simulink model "ofdm_txrx_supermimo_coded"  (Simulink 7.7)
settings: Solver=VariableStepDiscrete  SolverMode=SingleTasking  StartTime=0.0  StopTime=2*simOnly_simLength  RelTol=1e-3
PostLoadFcn:
  | ofdm_rx_supermimo_init
InitFcn:
  | ofdm_rx_supermimo_init
StartFcn:
  | ofdm_rx_supermimo_init
values from neighbouring files (symbols this model uses):
  ActionID_AFTransmit = 31    [ofdm_tx_supermimo_init.m]
  ActionID_Disabled = 0    [ofdm_tx_supermimo_init.m]
  ActionID_SetFlagA = 62    [ofdm_tx_supermimo_init.m]
  ActionID_SetFlagB = 61    [ofdm_tx_supermimo_init.m]
  BER_RAM_init_size = 2048    [ofdm_tx_supermimo_init.m]
  channel_h = [1 0; 1 0] * 0.3    [ofdm_tx_supermimo_init.m]
  CPLength = 16    [ofdm_tx_supermimo_init.m]
  longCorr_coef_i = [-4*real(LTS) 0]    [ofdm_rx_supermimo_init.m]
  longCorr_coef_q = [4*imag(LTS) 0]    [ofdm_rx_supermimo_init.m]
  max_CP_length = 16    [ofdm_tx_supermimo_init.m]
  max_num_baseRateSymbols = 31    [ofdm_tx_supermimo_init.m]
  max_num_subcarriers = 64    [ofdm_tx_supermimo_init.m]
  max_num_trainingSymbols = 15    [ofdm_tx_supermimo_init.m]
  max_numBytes = 16384    [ofdm_tx_supermimo_init.m]
  max_OFDM_symbols = 1023    [ofdm_tx_supermimo_init.m]
  modConstellation_qam16 = .75*[1 3 -1 -3]./3    [ofdm_tx_supermimo_init.m]
  modConstellation_qam64 = 0.875*[3 1 5 7 -3 -1 -5 -7]./7    [ofdm_tx_supermimo_init.m]
  modConstellation_qpsk = [1 -1]./sqrt(2)    [ofdm_tx_supermimo_init.m]
  numBaseRateSymbols = numBaseRateSymbols *2    [ofdm_tx_supermimo_init.m]
  numSubcarriers = 64    [ofdm_tx_supermimo_init.m]
  numTrainingSymbols = 2    [ofdm_tx_supermimo_init.m]
  pktTiming_count_payloadStart = pktTiming_count_lsStart + pktTiming_ls_num*pktTiming_ls_length    [ofdm_rx_supermimo_init.m]
  preamble = preamble_scale*[shortsyms_10 longsyms_2]    [ofdm_tx_supermimo_init.m]
  preamble_ROM = preamble_I_ROM + preamble_Q_ROM*2^16    [ofdm_tx_supermimo_init.m]
  RAM_init_size = max_numBytes    (= 16384)    [ofdm_tx_supermimo_init.m]
  RxDataScrambling_Seq = [40 198 78 63 82 173 102 245 48 111 172 115 147 230 216 93 72 65 62 2 205 242 122 90 128 83 105 97 73 10 5 252]    [ofdm_tx_supermimo_init.m]
  shortSymbol_time = shortSymbol_time(1:16).'    [ofdm_tx_supermimo_init.m]
  simOnly_numSamples = length(preamble)+( (numSubcarriers+CPLength)*(numTrainingSymbols + numBaseRateSymbols + numFullRateSymbols/2) )    [ofdm_tx_supermimo_init.m]
  train = [train train]    [ofdm_tx_supermimo_init.m]
  TxDataScrambling_Seq = [40 198 78 63 82 173 102 245 48 111 172 115 147 230 216 93 72 65 62 2 205 242 122 90 128 83 105 97 73 10 5 252]    [ofdm_tx_supermimo_init.m]
undefined symbols (not defined in the file or in its folder): AntA, AntB, BaseRate, Down, gcbh, numBuffs, numBytes, Up, xlBlockLoadCallback, xlGetNormalizedPeriod, xlipgetpartsetting
libraries: xbsIndex_r4 x5430
(part 1 of 49)
settings Model: Creator=murphpo  LastModifiedBy=murphpo
settings DataIOCC: LimitDataPoints=off  SaveOutput=off  SignalLogging=off  SaveTime=off
settings OptimizationCC: InlineParams=on  InitFltsAndDblsToZero=on
settings DebuggingCC: SaveWithParameterizedLinksMsg=none  StrictBusMsg=Warning

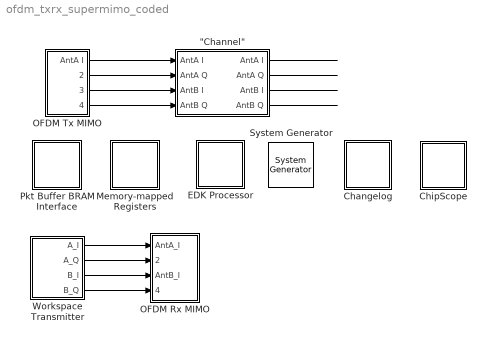
== system ofdm_txrx_supermimo_coded ==
  " System Generator" : xbsIndex_r4/ System Generator  Tag=genX  SystemSampleTime=-1  xilinxfamily=virtex6  part=xc6vlx130t  speed=-1  package=ff1156  synthesis_tool=XST  clock_wrapper=Clock Enables  directory=./OFDM_txrx_SG13_V6_v04_12bitIO  proj_type=Project Navigator  Synth_file=XST Defaults  Impl_file=ISE Defaults  testbench=off  simulink_period=1  sysclk_period=10  dcm_input_clock_period=100  incr_netlist=off  trim_vbits=Everywhere in SubSystem  dbl_ovrd=According to Block Masks  core_generation=According to Block Masks  run_coregen=off  deprecated_control=off  eval_field=0  block_type=sysgen
      settings: block_version=10.1.3
  "\"Channel\"" : Subsystem  mask{h=channel_h %[1 0; 0 0] * 0.65 % [1 0; 0 0] * 0.5, freqOffsetA=7.5e-4, freqOffsetB=0, awgnSNR=40, phNoise=-5000}
      mask prompts: h "2x2 Channel Matrix"; freqOffsetA "Freq Offset A (phase inc [0,1])"; freqOffsetB "Freq Offset B (phase inc [0,1])"; awgnSNR "SNR (dB)"; phNoise "Phase Noise (dBc @ 100Hz)"
      mask help: This block implements a simple static 2x2 channel. The one parameter sets the 2x2 channel matrix. h=[1 0;0 1] would be perfectly orthogonal channels. The norm of the matrix should be 1 to avoid overflow.
  Changelog : Subsystem
  ChipScope : Subsystem
  EDK Processor : Subsystem  LoadFcn=xlBlockLoadCallback(gcbh);  mask(Xilinx EDK Processor Block){mode=EDK pcore generation, xmp=, MemVisToProc=<qt bgcolor="#FFFFFF"><div><img src="C:/Xilinx/13.4/ISE_DS/ISE/sysgen/data/images/registerplus.gif"> &lt;&lt;FEC_Config&gt;&gt;<br></div><div><img src="C:/Xilinx/13.4/ISE_DS/ISE/sysgen/data/images/registerplus.gif"> &lt;&lt;Tx_OFDM_SymCounts&gt;&gt;<br></div><div><img src="C:/Xilinx/13.4/ISE_DS/ISE/sysgen/data/images/registerplus.gif"> &lt;&lt;Rx_OFDM_SymbolCounts&gt;&gt;<br></div><div><img src="C:/Xilinx/13.4/ISE_DS/ISE/sysgen/data/images/registerplus.gif"> &lt;&lt;TxRx_Pilots_Values&gt;&gt;<br></div><div><img src="C:/Xilinx/13.4/ISE_DS/ISE/sysgen/data/images/registerplus.gif"> &lt;&lt;TxRx_Pilots_Index&gt;&gt;<br></div><div><img src="C:/Xilinx/13.4/ISE_DS/ISE/sysgen/data/images/registerplus.gif"> &lt;&lt;TxRx_Interrupt_PktBuf_Ctrl&gt;&gt;<br></div><div><img src="C:/Xilinx/13.4/ISE_DS/ISE/sysgen/data/images/registerplus.gif"> &lt;&lt;TxRx_FFT_Scaling&gt;&gt;<br></div><div><img src="C:/Xilinx/13.4/ISE_DS/ISE/sysgen/data/images/registerplus.gif"> &lt;&lt;Tx_Start_Reset_Control&gt;&gt;<br></div><div><img src="C:/Xilinx/13.4/ISE_DS/ISE/sysgen/data/images/registerplus.gif"> &lt;&lt;Tx_ControlBits&gt;&gt;<br></div><div><img src="C:/Xilinx/13.4/ISE_DS/ISE/sysgen/data/images/registerplus.gif"> &lt;&lt;Tx_Delays&gt;&gt;<br></div><div><img src="C:/Xilinx/13.4/ISE_DS/ISE/sysgen/data/images/registerplus.gif"> &lt;&lt;Tx_Scaling&gt;&gt;<br></div><div><img src="C:/Xilinx/13.4/ISE_DS/ISE/sysgen/data/images/registerplus.gif"> &lt;&lt;pktDet_thresholds&gt;&gt;<br></div><div><img src="C:/Xilinx/13.4/ISE_DS/ISE/sysgen/data/images/registerplus.gif"> &lt;&lt;pktDet_durations&gt;&gt;<br></div><div><img src="C:/Xilinx/13.4/ISE_DS/ISE/sysgen/data/images/registerplus.gif"> &lt;&lt;pktDet_autoCorrParams&gt;&gt;<br></div><div><img src="C:/Xilinx/13.4/ISE_DS/ISE/sysgen/data/images/registerplus.gif"> &lt;&lt;pktDet_controlBits&gt;&gt;<br></div><div><img src="C:/Xilinx/13.4/ISE_DS/ISE/sysgen/data/images/registerplus.gif"> &lt;&lt;Rx_AF_TxScaling&gt;&gt;<br></div><div><img src="C:/Xilinx/13.4/ISE_DS/ISE/sysgen/data/images/registerplus.gif"> &lt;&lt;Rx_AF_Blanking&gt;&gt;<br></div><div><img src="C:/Xilinx/13.4/ISE_DS/ISE/sysgen/data/images/registerplus.gif"> &lt;&lt;Rx_PilotCalcParams&gt;&gt;<br></div><div><img src="C:/Xilinx/13.4/ISE_DS/ISE/sysgen/data/images/registerminus.gif"> &lt;&lt;Rx_Constellation_Scaling&gt;&gt;<br> <img src="C:/Xilinx/13.4/ISE_DS/ISE/sysgen/data/images/parameters.gif"> Type : Fix_32_0<br> <img src="C:/Xilinx/13.4/ISE_DS/ISE/sysgen/data/images/parameters.gif"> Addr : 0x80008048<br></div><div><img src="C:/Xilinx/13.4/ISE_DS/ISE/sysgen/data/images/registerplus.gif"> &lt;&lt;Rx_PktDet_Delay&gt;&gt;<br></div><div><img src="C:/Xilinx/13.4/ISE_DS/ISE/sysgen/data/images/registerplus.gif"> &lt;&lt;Rx_ChanEst_MinMag&gt;&gt;<br></div><div><img src="C:/Xilinx/13.4/ISE_DS/ISE/sysgen/data/images/registerplus.gif"> &lt;&lt;Rx_pktByteNums&gt;&gt;<br></div><div><img src="C:/Xilinx/13.4/ISE_DS/ISE/sysgen/data/images/registerplus.gif"> &lt;&lt;Rx_FixedPktLen&gt;&gt;<br></div><div><img src="C:/Xilinx/13.4/ISE_DS/ISE/sysgen/data/images/registerplus.gif"> &lt;&lt;Rx_PktDet_LongCorr_Thresholds&gt;&gt;<br></div><div><img src="C:/Xilinx/13.4/ISE_DS/ISE/sysgen/data/images/registerplus.gif"> &lt;&lt;Rx_PktDet_LongCorr_Params&gt;&gt;<br></div><div><img src="C:/Xilinx/13.4/ISE_DS/ISE/sysgen/data/images/registerplus.gif"> &lt;&lt;Rx_coarseCFO_correction&gt;&gt;<br></div><div><img src="C:/Xilinx/13.4/ISE_DS/ISE/sysgen/data/images/registerplus.gif"> &lt;&lt;Rx_PreCFO_PilotCalcCorrection&gt;&gt;<br></div><div><img src="C:/Xilinx/13.4/ISE_DS/ISE/sysgen/data/images/registerplus.gif"> &lt;&lt;Rx_PreCFO_Options&gt;&gt;<br></div><div><img src="C:/Xilinx/13.4/ISE_DS/ISE/sysgen/data/images/registerplus.gif"> &lt;&lt;Rx_ControlBits&gt;&gt;<br></div><div><img src="C:/Xilinx/13.4/ISE_DS/ISE/sysgen/data/images/registerplus.gif"> &lt;&lt;TxRx_AutoReply_Action3&gt;&gt;<br></div><div><img src="C:/Xilinx/13.4/ISE_DS/ISE/sysgen/data/images/registerplus.gif"> &lt;&lt;TxRx_AutoReply_Action2&gt;&gt;<br></div><div><img src="C:/Xilinx/13.4/ISE_DS/ISE/sysgen/data/images/registerplus.gif"> &lt;&lt;TxRx_AutoReply_Action1&gt;&gt;<br></div><div><img src="C:/Xilinx/13.4/ISE_DS/ISE/sysgen/data/images/registerplus.gif"> &lt;&lt;TxRx_AutoReply_Action0&gt;&gt;<br></div><div><img src="C:/Xilinx/13.4/ISE_DS/ISE/sysgen/data/images/registerplus.gif"> &lt;&lt;TxRx_AutoReply_Match5&gt;&gt;<br></div><div><img src="C:/Xilinx/13.4/ISE_DS/ISE/sysgen/data/images/registerplus.gif"> &lt;&lt;TxRx_AutoReply_Match4&gt;&gt;<br></div><div><img src="C:/Xilinx/13.4/ISE_DS/ISE/sysgen/data/images/registerplus.gif"> &lt;&lt;TxRx_AutoReply_Match3&gt;&gt;<br></div><div><img src="C:/Xilinx/13.4/ISE_DS/ISE/sysgen/data/images/registerplus.gif"> &lt;&lt;TxRx_AutoReply_Match2&gt;&gt;<br></div><div><img src="C:/Xilinx/13.4/ISE_DS/ISE/sysgen/data/images/registerplus.gif"> &lt;&lt;TxRx_AutoReply_Action5&gt;&gt;<br></div><div><img src="C:/Xilinx/13.4/ISE_DS/ISE/sysgen/data/images/registerplus.gif"> &lt;&lt;TxRx_AutoReply_Action4&gt;&gt;<br></div><div><img src="C:/Xilinx/13.4/ISE_DS/ISE/sysgen/data/images/registerplus.gif"> &lt;&lt;TxRx_AutoReply_Match1&gt;&gt;<br></div><div><img src="C:/Xilinx/13.4/ISE_DS/ISE/sysgen/data/images/registerplus.gif"> &lt;&lt;TxRx_AutoReply_Match0&gt;&gt;<br></div><div><img src="C:/Xilinx/13.4/ISE_DS/ISE/sysgen/data/images/registerplus.gif"> &lt;&lt;midPacketRSSI&gt;&gt;<br></div><div><img src="C:/Xilinx/13.4/ISE_DS/ISE/sysgen/data/images/registerplus.gif"> &lt;&lt;Tx_PktRunning&gt;&gt;<br></div><div><img src="C:/Xilinx/13.4/ISE_DS/ISE/sysgen/data/images/registerplus.gif"> &lt;&lt;Rx_pktDetEventCount&gt;&gt;<br></div><div><img src="C:/Xilinx/13.4/ISE_DS/ISE/sysgen/data/images/registerplus.gif"> &lt;&lt;Rx_coarseCFOest&gt;&gt;<br></div><div><img src="C:/Xilinx/13.4/ISE_DS/ISE/sysgen/data/images/registerplus.gif"> &lt;&lt;Rx_pilotCFOest&gt;&gt;<br></div><div><img src="C:/Xilinx/13.4/ISE_DS/ISE/sysgen/data/images/registerplus.gif"> &lt;&lt;Rx_pktDone_interruptStatus&gt;&gt;<br></div><div><img src="C:/Xilinx/13.4/ISE_DS/ISE/sysgen/data/images/registerplus.gif"> &lt;&lt;Rx_BER_TotalBits&gt;&gt;<br></div><div><img src="C:/Xilinx/13.4/ISE_DS/ISE/sysgen/data/images/registerplus.gif"> &lt;&lt;Rx_BER_Errors&gt;&gt;<br></div><div><img src="C:/Xilinx/13.4/ISE_DS/ISE/sysgen/data/images/registerplus.gif"> &lt;&lt;Rx_Gains&gt;&gt;<br></div><div><img src="C:/Xilinx/13.4/ISE_DS/ISE/sysgen/data/images/registerplus.gif"> &lt;&lt;pktDet_status&gt;&gt;<br></div><div><img src="C:/Xilinx/13.4/ISE_DS/ISE/sysgen/data/images/memoryplus.gif"> &lt;&lt;TxModulation&gt;&gt;<br></div><div><img src="C:/Xilinx/13.4/ISE_DS/ISE/sysgen/data/images/memoryplus.gif"> &lt;&lt;TxHeaderTranslate&gt;&gt;<br></div><div><img src="C:/Xilinx/13.4/ISE_DS/ISE/sysgen/data/images/memoryplus.gif"> &lt;&lt;ChannelEstimates&gt;&gt;<br></div><div><img src="C:/Xilinx/13.4/ISE_DS/ISE/sysgen/data/images/memoryplus.gif"> &lt;&lt;RxModulation&gt;&gt;<br></div><div><img src="C:/Xilinx/13.4/ISE_DS/ISE/sysgen/data/images/memoryplus.gif"> &lt;&lt;EVM_perSym&gt;&gt;<br></div><div><img src="C:/Xilinx/13.4/ISE_DS/ISE/sysgen/data/images/memoryplus.gif"> &lt;&lt;EVM_perSC&gt;&gt;<br></div><div><img src="C:/Xilinx/13.4/ISE_DS/ISE/sysgen/data/images/memoryplus.gif"> &lt;&lt;PktBufFreqOffsets&gt;&gt;<br></div></qt>, AvailableMemories=<empty>, portInterfaceTable={'exposed'=>[],'portdir'=>[],'portname'=>[],'shortname'=>[]}, bus_type_sgadvanced=, bus_type=PLB, baseaddr=0x80000000, baseaddr_lock_sgadvanced=, baseaddr_lock=off, dual_clock_sgadvanced=, dual_clock=on, reg_readback_sgadvanced=, reg_readback=on, ucf_file=, inheritDeviceType_sgadvanced=, inheritDeviceType=off, elf_file=, codebug_sgadvanced=, codebug=on, clock_name=plb, internalPortList={}, resetPolarity=0, memxtable=|, procinfo={}, memmapdirty=off, blockname=, xpsintstyle=default, has_advanced_control=0, sggui_pos=-1,-1,-1,-1, block_type=edkprocessor, block_version=2.7, sg_icon_stat=44,44,-1,-1,white,blue,0,07734,right,,[ ],[ ], sg_mask_display=|, sg_list_contents={'table'=>{'AvailableMemories'=>'popup(<empty>)'}}, sg_blockgui_xml=}
      mask memxtable=|
      | {'mladdr'=>[0.00000000000000000,1.00000000000000000,2.00000000000000000,3.00000000000000000,4.00000000000000000,5.00000000000000000,6.00000000000000000,7.00000000000000000,8.00000000000000000,9.00000000000000000,10.00000000000000000,11.00000000000000000,12.00000000000000000,13.00000000000000000,14.00000000000000000,15.00000000000000000,16.00000000000000000,17.00000000000000000,18.00000000000000000,19.00000000000000000,20.00000000000000000,21.00000000000000000,22.00000000000000000,23.00000000000000000,24.00000000000000000,25.00000000000000000,26.00000000000000000,27.00000000000000000,28.00000000000000000,29.00000000000000000,30.00000000000000000,31.00000000000000000,32.00000000000000000,33.00000000000000000,34.00000000000000000,35.00000000000000000,36.00000000000000000,37.00000000000000000,38.00000000000000000,39.00000000000000000,40.00000000000000000,0.00000000000000000,1.00000000000000000,2.00000000000000000,3.00000000000000000,4.00000000000000000,5.00000000000000000,6.00000000000000000,7.00000000000000000,8.00000000000000000,9.00000000000000000,-1.00000000000000000,-1.00000000000000000,-1.00000000000000000,-1.00000000000000000,-1.00000000000000000,-1.00000000000000000,-1.00000000000000000],'mlist'=>['ofdm_txrx_supermimo_coded/Memory-mapped
      | Registers/TxRx Registers/From Register8','ofdm_txrx_supermimo_coded/Memory-mapped
      | Registers/TxRx Registers/From Register7','ofdm_txrx_supermimo_coded/Memory-mapped
      | Registers/TxRx Registers/From Register6','ofdm_txrx_supermimo_coded/Memory-mapped
      | Registers/TxRx Registers/From Register5','ofdm_txrx_supermimo_coded/Memory-mapped
      | Registers/TxRx Registers/From Register4','ofdm_txrx_supermimo_coded/Memory-mapped
      | Registers/TxRx Registers/From Register3','ofdm_txrx_supermimo_coded/Memory-mapped
      | Registers/TxRx Registers/From Register2','ofdm_txrx_supermimo_coded/Memory-mapped
      | Registers/Tx Registers/From Register3','ofdm_txrx_supermimo_coded/Memory-mapped
      | Registers/Tx Registers/From Register2','ofdm_txrx_supermimo_coded/Memory-mapped
      | Registers/Tx Registers/From Register10','ofdm_txrx_supermimo_coded/Memory-mapped
      | Registers/Tx Registers/From Register1','ofdm_txrx_supermimo_coded/Memory-mapped
      | Registers/Rx Registers/Pkt Detector Registers/From Register4','ofdm_txrx_supermimo_coded/Memory-mapped
      | Registers/Rx Registers/Pkt Detector Registers/From Register3','ofdm_txrx_supermimo_coded/Memory-mapped
      | Registers/Rx Registers/Pkt Detector Registers/From Register2','ofdm_txrx_supermimo_coded/Memory-mapped
      | Registers/Rx Registers/Pkt Detector Registers/From Register1','ofdm_txrx_supermimo_coded/Memory-mapped
      | Registers/Rx Registers/From Register8','ofdm_txrx_supermimo_coded/Memory-mapped
      | Registers/Rx Registers/From Register7','ofdm_txrx_supermimo_coded/Memory-mapped
      | Registers/Rx Registers/From Register6','ofdm_txrx_supermimo_coded/Memory-mapped
      | Registers/Rx Registers/From Register5','ofdm_txrx_supermimo_coded/Memory-mapped
      | Registers/Rx Registers/From Register4','ofdm_txrx_supermimo_coded/Memory-mapped
      | Registers/Rx Registers/From Register3','ofdm_txrx_supermimo_coded/Memory-mapped
      | Registers/Rx Registers/From Register2','ofdm_txrx_supermimo_coded/Memory-mapped
      | Registers/Rx Registers/From Register16','ofdm_txrx_supermimo_coded/Memory-mapped
      | Registers/Rx Registers/From Register14','ofdm_txrx_supermimo_coded/Memory-mapped
      | Registers/Rx Registers/From Register13','ofdm_txrx_supermimo_coded/Memory-mapped
      | Registers/Rx Registers/From Register12','ofdm_txrx_supermimo_coded/Memory-mapped
      | Registers/Rx Registers/From Register11','ofdm_txrx_supermimo_coded/Memory-mapped
      | Registers/Rx Registers/From Register10','ofdm_txrx_supermimo_coded/Memory-mapped
      | Registers/Rx Registers/From Register','ofdm_txrx_supermimo_coded/Memory-mapped
      | Registers/AutoReply Registers/From Register9','ofdm_txrx_supermimo_coded/Memory-mapped
      | Registers/AutoReply Registers/From Register8','ofdm_txrx_supermimo_coded/Memory-mapped
      | Registers/AutoReply Registers/From Register7','ofdm_txrx_supermimo_coded/Memory-mapped
      | Registers/AutoReply Registers/From Register6','ofdm_txrx_supermimo_coded/Memory-mapped
      | Registers/AutoReply Registers/From Register5','ofdm_txrx_supermimo_coded/Memory-mapped
      | Registers/AutoReply Registers/From Register4','ofdm_txrx_supermimo_coded/Memory-mapped
      | Registers/AutoReply Registers/From Register3','ofdm_txrx_supermimo_coded/Memory-mapped
      | Registers/AutoReply Registers/From Register2','ofdm_txrx_supermimo_coded/Memory-mapped
      | Registers/AutoReply Registers/From Register11','ofdm_txrx_supermimo_coded/Memory-mapped
      | Registers/AutoReply Registers/From Register10','ofdm_txrx_supermimo_coded/Memory-mapped
      | Registers/AutoReply Registers/From Register1','ofdm_txrx_supermimo_coded/Memory-mapped
      | Registers/AutoReply Registers/From Register','ofdm_txrx_supermimo_coded/OFDM Rx MIMO/Packet_Detection/RSSI-based PktDet/Mid-Packet
      | RSSI Register/To Register2','ofdm_txrx_supermimo_coded/Memory-mapped
      | Registers/Tx Registers/To Register7','ofdm_txrx_supermimo_coded/Memory-mapped
      | Registers/Rx Registers/To Register6','ofdm_txrx_supermimo_coded/Memory-mapped
      | Registers/Rx Registers/To Register5','ofdm_txrx_supermimo_coded/Memory-mapped
      | Registers/Rx Registers/To Register4','ofdm_txrx_supermimo_coded/Memory-mapped
      | Registers/Rx Registers/To Register3','ofdm_txrx_supermimo_coded/Memory-mapped
      | Registers/Rx Registers/To Register2','ofdm_txrx_supermimo_coded/Memory-mapped
      | Registers/Rx Registers/To Register1','ofdm_txrx_supermimo_coded/Memory-mapped
      | Registers/Rx Registers/To Register','ofdm_txrx_supermimo_coded/Memory-mapped
      | Registers/Rx Registers/Pkt Detector Registers/To Register','ofdm_txrx_supermimo_coded/OFDM Tx MIMO/Training_Data/FlexibleMod/control/Tx Modulation/Shared Memory for
      | Modulation Masks/Shared Memory','ofdm_txrx_supermimo_coded/OFDM Tx MIMO/Training_Data/FlexibleMod/PktBuffer_CRC1/Packet Buffer/AutoTx
      | Header Translation/Shared Memory','ofdm_txrx_supermimo_coded/OFDM Rx MIMO/FFT & Chan Est/Channel Estimation
      | & Pilot Processing/Chan Est Buffer
      | & Mag Calc/Shared Memory','ofdm_txrx_supermimo_coded/OFDM Rx MIMO/Equalizer & Packetizer/Packet_Constructor/Modulation RAM/Modulation Masks/Shared Memory','ofdm_txrx_supermimo_coded/OFDM Rx MIMO/Equalizer & Packetizer/Packet_Constructor/EVM Calc/EVM per Symbol/Shared Memory','ofdm_txrx_supermimo_coded/OFDM Rx MIMO/Equalizer & Packetizer/Packet_Constructor/EVM Calc/EVM per Subcarrier/Shared Memory','ofdm_txrx_supermimo_coded/OFDM Rx MIMO/Coarse Freq Correction/PreSpin CFO Sel/Shared Memory'],'mlname'=>['\\'FEC_Config\\'','\\'Tx_OFDM_SymCounts\\'','\\'Rx_OFDM_SymbolCounts\\'','\\'TxRx_Pilots_Values\\'','\\'TxRx_Pilots_Index\\'','\\'TxRx_Interrupt_PktBuf_Ctrl\\'','\\'TxRx_FFT_Scaling\\'','\\'Tx_Start_Reset_Control\\'','\\'Tx_ControlBits\\'','\\'Tx_Delays\\'','\\'Tx_Scaling\\'','\\'pktDet_thresholds\\'','\\'pktDet_durations\\'','\\'pktDet_autoCorrParams\\'','\\'pktDet_controlBits\\'','\\'Rx_AF_TxScaling\\'','\\'Rx_AF_Blanking\\'','\\'Rx_PilotCalcParams\\'','\\'Rx_Constellation_Scaling\\'','\\'Rx_PktDet_Delay\\'','\\'Rx_ChanEst_MinMag\\'','\\'Rx_pktByteNums\\'','\\'Rx_FixedPktLen\\'','\\'Rx_PktDet_LongCorr_Thresholds\\'','\\'Rx_PktDet_LongCorr_Params\\'','\\'Rx_coarseCFO_correction\\'','\\'Rx_PreCFO_PilotCalcCorrection\\'','\\'Rx_PreCFO_Options\\'','\\'Rx_ControlBits\\'','\\'TxRx_AutoReply_Action3\\'','\\'TxRx_AutoReply_Action2\\'','\\'TxRx_AutoReply_Action1\\'','\\'TxRx_AutoReply_Action0\\'','\\'TxRx_AutoReply_Match5\\'','\\'TxRx_AutoReply_Match4\\'','\\'TxRx_AutoReply_Match3\\'','\\'TxRx_AutoReply_Match2\\'','\\'TxRx_AutoReply_Action5\\'','\\'TxRx_AutoReply_Action4\\'','\\'TxRx_AutoReply_Match1\\'','\\'TxRx_AutoReply_Match0\\'','\\'midPacketRSSI\\'','\\'Tx_PktRunning\\'','\\'Rx_pktDetEventCount\\'','\\'Rx_coarseCFOest\\'','\\'Rx_pilotCFOest\\'','\\'Rx_pktDone_interruptStatus\\'','\\'Rx_BER_TotalBits\\'','\\'Rx_BER_Errors\\'','\\'Rx_Gains\\'','\\'pktDet_status\\'','\\'TxModulation\\'','\\'TxHeaderTranslate\\'','\\'ChannelEstimates\\'','\\'RxModulation\\'','\\'EVM_perSym\\'','\\'EVM_perSC\\'','\\'PktBufFreqOffsets\\''],'mlstate'=>[0.00000000000000000,0.00000000000000000,0.00000000000000000,0.00000000000000000,0.00000000000000000,0.00000000000000000,0.00000000000000000,0.00000000000000000,0.00000000000000000,0.00000000000000000,0.00000000000000000,0.00000000000000000,0.00000000000000000,0.00000000000000000,0.00000000000000000,0.00000000000000000,0.00000000000000000,0.00000000000000000,1.00000000000000000,0.00000000000000000,0.00000000000000000,0.00000000000000000,0.00000000000000000,0.00000000000000000,0.00000000000000000,0.00000000000000000,0.00000000000000000,0.00000000000000000,0.00000000000000000,0.00000000000000000,0.00000000000000000,0.00000000000000000,0.00000000000000000,0.00000000000000000,0.00000000000000000,0.00000000000000000,0.00000000000000000,0.00000000000000000,0.00000000000000000,0.00000000000000000,0.00000000000000000,0.00000000000000000,0.00000000000000000,0.00000000000000000,0.00000000000000000,0.00000000000000000,0.00000000000000000,0.00000000000000000,0.00000000000000000,0.00000000000000000,0.00000000000000000,0.00000000000000000,0.00000000000000000,0.00000000000000000,0.00000000000000000,0.00000000000000000,0.00000000000000000,0.00000000000000000]}
      mask sg_mask_display=|
      | fprintf('','COMMENT: begin icon graphics');
      | patch([0 44 44 0 0 ],[0 0 44 44 0 ],[0.77 0.82 0.91 ]);
      | plot([0 44 44 0 0 ],[0 0 44 44 0 ]);
      | patch([8.65 17.32 23.32 29.32 35.32 23.32 14.65 8.65 ],[28.66 28.66 34.66 28.66 34.66 34.66 34.66 28.66 ],[1 1 1 ]);
      | patch([14.65 23.32 17.32 8.65 14.65 ],[22.66 22.66 28.66 28.66 22.66 ],[0.931 0.946 0.973 ]);
      | patch([8.65 17.32 23.32 14.65 8.65 ],[16.66 16.66 22.66 22.66 16.66 ],[1 1 1 ]);
      | patch([14.65 35.32 29.32 23.32 17.32 8.65 14.65 ],[10.66 10.66 16.66 10.66 16.66 16.66 10.66 ],[0.931 0.946 0.973 ]);
      | fprintf('','COMMENT: end icon graphics');
      | fprintf('','COMMENT: begin icon text');
      | fprintf('','COMMENT: end icon text');
      mask prompts: mode "Configure Processor for"; xmp "XPS Project"; MemVisToProc ""; AvailableMemories "Available Memories"; portInterfaceTable ""; bus_type_sgadvanced ""; bus_type "Bus Type"; baseaddr "Base Address"; baseaddr_lock_sgadvanced ""; baseaddr_lock "Lock"; dual_clock_sgadvanced ""; dual_clock "Dual Clocks"; reg_readback_sgadvanced ""; reg_readback "Register Read-Back"; ucf_file "Constraint File"; inheritDeviceType_sgadvanced ""; inheritDeviceType "Inherit Device Type"; elf_file "Initial Program"; codebug_sgadvanced ""; codebug "Enable Co-Debug with Xilinx SDK (Beta)"; clock_name ""; internalPortList ""; resetPolarity ""; memxtable ""; procinfo ""; memmapdirty ""; blockname ""; xpsintstyle ""; has_advanced_control ""; sggui_pos ""; block_type ""; block_version ""; sg_icon_stat ""; sg_mask_display ""; sg_list_contents ""; sg_blockgui_xml ""
      mask help: Xilinx EDK Processor
      mask init:
      | try
      |   tmp_gcb = gcb;
      |   tmp_gcbh = gcbh;
      |   if (strcmp('SysGenIndex',get_param(bdroot(tmp_gcbh),'tag')) && ~isempty(regexp(bdroot(tmp_gcb), '^xbs', 'once')))
      |     return;
      |   end;
      |   xlMungeMaskParams;
      | 
      |   block_type='edkprocessor';
      | 
      |   serialized_declarations = '{''block_type''=>''String''}';
      |   xledkprocessor_init();
      |   ptable_ = xlblockprep(get_param(tmp_gcb, 'MaskWSVariables'));
      |   try
      |     xlBlockMoveCallback(tmp_gcbh);
      |   catch 
      |      clear global xl_updateicon_recursion_guard;
      |   end;
      | catch
      |   global dbgsysgen;
      |   if(~isempty(dbgsysgen) && dbgsysgen)
      |     e = regexprep(lasterr, '\n', '\nError: ');
      |     disp(['Error: While running MaskInit code on block ' tmp_gcb ': ' e]);
      |     error(e);
      |   end
      | end
      settings: CopyFcn=xlProcBlockCopyCallback(gcbh);xlBlockMoveCallback(gcbh);  DeleteFcn=xlDestroyGui(gcbh);  ModelCloseFcn=xlDestroyGui(gcbh);  PreSaveFcn=xlBlockPreSaveCallback(gcbh);  PostSaveFcn=xlBlockPostSaveCallback(gcbh);  DestroyFcn=xlDestroyGui(gcbh);  OpenFcn=bh=gcbh;xlProcBlockCallbacks('populatesharedmemorylistbox',bh);xlOpenGui(bh, 'edkprocessor_gui.xml', @xlProcBlockEnablement, @xlProcBlockAction);  CloseFcn=xlDestroyGui(gcbh);  MoveFcn=xlBlockMoveCallback(gcbh);
      mask Help: eval('');xlDoc('-book','sysgen','-topic','EDK_Processor');
      mask StyleString: popup(EDK pcore generation|HDL netlisting),edit,edit,edit,edit,edit,popup(AXI|PLB),edit,edit,checkbox,edit,checkbox,edit,checkbox,edit,edit,checkbox,edit,edit,checkbox,edit,edit,edit,edit,edit,edit,edit,edit,edit,edit,edit,edit,edit,edit,edit,edit
      mask Display:
      | fprintf('','COMMENT: begin icon graphics');
      | patch([0 44 44 0 0 ],[0 0 44 44 0 ],[0.77 0.82 0.91 ]);
      | plot([0 44 44 0 0 ],[0 0 44 44 0 ]);
      | patch([8.65 17.32 23.32 29.32 35.32 23.32 14.65 8.65 ],[28.66 28.66 34.66 28.66 34.66 34.66 34.66 28.66 ],[1 1 1 ]);
      | patch([14.65 23.32 17.32 8.65 14.65 ],[22.66 22.66 28.66 28.66 22.66 ],[0.931 0.946 0.973 ]);
      | patch([8.65 17.32 23.32 14.65 8.65 ],[16.66 16.66 22.66 22.66 16.66 ],[1 1 1 ]);
      | patch([14.65 35.32 29.32 23.32 17.32 8.65 14.65 ],[10.66 10.66 16.66 10.66 16.66 16.66 10.66 ],[0.931 0.946 0.973 ]);
      | fprintf('','COMMENT: end icon graphics');
      | fprintf('','COMMENT: begin icon text');
      | disp('');
      | 
      | fprintf('','COMMENT: end icon text');
  Memory-mapped Registers : Subsystem
  OFDM Rx MIMO : Subsystem
  OFDM Tx MIMO : Subsystem
  Pkt Buffer BRAM Interface : Subsystem
  Workspace Transmitter : Subsystem
  wires:
  "\"Channel\""/1(" AntA I") -> ?
  "\"Channel\""/2(" AntA Q") -> ?
  "\"Channel\""/3(" AntB I") -> ?
  "\"Channel\""/4(" AntB Q") -> ?
  OFDM Tx MIMO/1(AntA I) -> "\"Channel\""/1(AntA I)
  OFDM Tx MIMO/2(AntA Q) -> "\"Channel\""/2(AntA Q)
  OFDM Tx MIMO/3(AntB I) -> "\"Channel\""/3(AntB I)
  OFDM Tx MIMO/4(AntB Q) -> "\"Channel\""/4(AntB Q)
  Workspace Transmitter/1(A_I) -> OFDM Rx MIMO/1(AntA_I)
  Workspace Transmitter/2(A_Q) -> OFDM Rx MIMO/2(AntA_Q)
  Workspace Transmitter/3(B_I) -> OFDM Rx MIMO/3(AntB_I)
  Workspace Transmitter/4(B_Q) -> OFDM Rx MIMO/4(AntB_Q)

== system ofdm_txrx_supermimo_coded/"Channel" ==
  note: Phase Noise
      | blocks were here
  note: AWGN
      | blocks were here
  AntA I : Inport
  AntA Q : Inport  Port=2
  AntB I : Inport  Port=3
  AntB Q : Inport  Port=4
  Add : Sum  Inputs=+-
  Add1 : Sum  Inputs=+-
  Complex to Magnitude-Angle{,1..3} (4 alike) : ComplexToMagnitudeAngle
  Complex to Real-Imag1 : ComplexToRealImag
  Complex to Real-Imag2 : ComplexToRealImag
  Delays : Subsystem
  Freq Offset : Subsystem
  Gain : Gain
  Gain1 : Gain
  "MIMO \"Channel\"" : Subsystem
  Real-Imag to Complex : RealImagToComplex
  Real-Imag to Complex1 : RealImagToComplex
  Zero-Order Hold{,1..3} (4 alike) : ZeroOrderHold
  " AntA I" : Outport
  " AntA Q" : Outport  Port=2
  " AntB I" : Outport  Port=3
  " AntB Q" : Outport  Port=4
  wires:
  AntA I/1 -> Zero-Order Hold/1
  AntA Q/1 -> Zero-Order Hold1/1
  AntB I/1 -> Zero-Order Hold2/1
  AntB Q/1 -> Zero-Order Hold3/1
  Complex to Real-Imag1/1 -> " AntA I"/1
  Complex to Real-Imag1/2 -> " AntA Q"/1
  Complex to Real-Imag2/1 -> " AntB I"/1
  Complex to Real-Imag2/2 -> " AntB Q"/1
  Gain/1 -> Complex to Magnitude-Angle/1, Complex to Magnitude-Angle1/1, Complex to Real-Imag1/1
  Gain1/1 -> Complex to Magnitude-Angle2/1, Complex to Magnitude-Angle3/1, Complex to Real-Imag2/1
  Real-Imag to Complex/1 -> Delays/1(In1)
  Real-Imag to Complex1/1 -> Delays/2(In2)
  Zero-Order Hold1/1 -> Real-Imag to Complex/2
  Zero-Order Hold2/1 -> Real-Imag to Complex1/1
  Zero-Order Hold3/1 -> Real-Imag to Complex1/2
  Zero-Order Hold/1 -> Real-Imag to Complex/1
  Complex to Magnitude-Angle/2 -> Add/1
  Complex to Magnitude-Angle1/2 -> Add/2
  Complex to Magnitude-Angle2/2 -> Add1/1
  Complex to Magnitude-Angle3/2 -> Add1/2
  Delays/1(Out1) -> Freq Offset/1(In1)
  Delays/2(Out2) -> Freq Offset/2(In2)
  "MIMO \"Channel\""/1(Out1) -> Gain/1
  "MIMO \"Channel\""/2(Out2) -> Gain1/1
  Freq Offset/1(Out1) -> "MIMO \"Channel\""/1(In1)
  Freq Offset/2(Out2) -> "MIMO \"Channel\""/2(In2)

== system ofdm_txrx_supermimo_coded/Changelog ==
  note: Export 4.00.a:
      | -First atempt at Sysgen 13.4 export
      |   -Replaced deprecated FFT/DDS/sin-cos cores
      |   -Updated indetermenite detector locations
      |   -Added bbReplace.m script to work around black box bug
      |   -Replaced old register wrapper subsystems with actual To/From regs
      |      (hoping "register readback" actually works)
      |
      | Export 4.00.b
      | -Reapplied read-before-write for chan ests used by pilot phase calc
      | -Updaed precision through autoCorr det (old values had lots of useless integer bits)
      | -Reconnected pilot atan block (was effecively disabled in 4.00.a)
      |
      | Export 4.00.c
      | -Removed artificial CFO (accidentally left in from debugging)
      | -Added BRAM_En output (always 1) to PORTB BUS_IF, so XPS stops complaining about non-BUS connecting in MHS
      |
      | Export 4.00.d
      | -Substituted fec_decoder.v for post-xst model provided by Xilinx tech support, to work around MATLAB crash during sim
      |
      | Export 4.00.e
      | -Sim-muxed fec_decoder, to use source HDL for export but sim HDL for simulation
      |
      | Export 4.01.a
      | -First export for V6; replaced unsupported cores, un-set saturation in EVM calc accumulator (not supported in v6)
      |   -Observed in hardware that the CORDIC divider doesn't work, at all; later reproduced this in a divider-only model
      |
      | Export 4.01.b
      | -Replaced CORDIC divdider with similarly-spec'd Divider Generator 3.0 core
      |
      | Export 4.01.c
      | -Fixed data type at div output to re-scale EQ output to match old div design
      |
      | Export 4.01.d
      | -Added lots of precision to autocorr pkt det. Hard-vs-soft mult versions were different precisions already, neither working very well
      |   for especially large or small I/Q
      | -Added min-duration for autoCorr pktdet (6 MSB of existing pkt det durations register)
      |
      | Export 4.01.e
      |  -Changed ADC/DAC gateways to Fix12_11 for compatibility with WARP v3 template project
      |  -Added pktDet signals as triggers for chipscope
  Through PHY 3.00i : Subsystem
  Through PHY 3.00u : Subsystem
  Through PHY 3.01f : Subsystem
  Through PHY 3.01x : Subsystem
  Through refdes14.0 : Subsystem

== system ofdm_txrx_supermimo_coded/ChipScope ==
  ChipScope1 : xbsIndex_r4/ChipScope  num_trig_ports=7  current_port=4  show_trig_port=0  match_units=1  match_type=Basic with edges  data_is_trigger=off  num_data_ports=20  data_depth=4096  SRL16s=on  add_bufg=on  match_type_t0=2  match_type_t1=2  match_type_t2=2  match_type_t3=2  match_type_t4=2  match_type_t5=1  match_type_t6=1  match_type_t7=1  match_type_t8=1  match_type_t9=1  match_type_t10=1  match_type_t11=1  match_type_t12=1  match_type_t13=1  match_type_t14=1  match_type_t15=1  match_units_t0=1  match_units_t1=1  match_units_t2=1  match_units_t3=1  match_units_t4=1  match_units_t5=1  match_units_t6=1  match_units_t7=1  match_units_t8=1  match_units_t9=1  match_units_t10=1  match_units_t11=1  match_units_t12=1  match_units_t13=1  match_units_t14=1  match_units_t15=1  dbl_ovrd=0  user_scan_chain=USER1  block_type=chipscope
      settings: infoedit="Enables run-time debugging and verification of signals by inserting ChipScope Pro ICON and ILA cores.<br><br>  Restrictions: <br>Only one ChipScope core can be instantiated in a System Generator design. A design or subsystem containing a ChipScope block must have at least one output port."  block_version=VER_STRING_GOES_HERE
  Constant1 : Constant  Value=0
  Convert1 : xbsIndex_r4/Convert  gui_display_data_type=Fixed-point  arith_type="Signed  (2's comp)"  n_bits=14  bin_pt=12  float_type=Single  exp_bits=8  fraction_bits=24  quantization=Truncate  overflow=Saturate  en=off  latency=0  dbl_ovrd=off  pipeline=off  block_type=convert
      settings: xl_area=[0 0 0 0 0 0 0]  block_version=VER_STRING_GOES_HERE
  Convert2 : xbsIndex_r4/Convert  gui_display_data_type=Fixed-point  arith_type=Unsigned  n_bits=14  bin_pt=0  float_type=Single  exp_bits=8  fraction_bits=24  quantization=Truncate  overflow=Wrap  en=off  latency=0  dbl_ovrd=off  pipeline=off  block_type=convert
      settings: xl_area=[0 0 0 0 0 0 0]  block_version=VER_STRING_GOES_HERE
  Counter1 : xbsIndex_r4/Counter  cnt_type=Free Running  cnt_to=Inf  operation=Up  start_count=0  cnt_by_val=1  arith_type=Unsigned  n_bits=2  bin_pt=0  load_pin=off  rst=off  en=off  explicit_period=on  period=1  dbl_ovrd=off  use_behavioral_HDL=off  implementation=Fabric  use_rpm=on  block_type=counter
      settings: xl_area=[5 10 0 10 0 0 0]  block_version=VER_STRING_GOES_HERE
  Slice1 : xbsIndex_r4/Slice  nbits=14  boolean_output=off  mode=Lower Bit Location + Width  bit1=0  base1=MSB of Input  bit0=4  base0=LSB of Input  dbl_ovrd=off  block_type=slice
      settings: infoedit="Extracts a given range of bits from each input sample and presents it at the output.  The output type is ordinarily unsigned with binary point at zero, but can be Boolean when the slice is one bit wide.<P><P>Hardware notes: In hardware this block costs nothing."  block_version=VER_STRING_GOES_HERE
  Slice2 : xbsIndex_r4/Slice  nbits=1  boolean_output=off  mode=Upper Bit Location + Width  bit1=0  base1=MSB of Input  bit0=0  base0=LSB of Input  dbl_ovrd=off  block_type=slice
      settings: infoedit="Extracts a given range of bits from each input sample and presents it at the output.  The output type is ordinarily unsigned with binary point at zero, but can be Boolean when the slice is one bit wide.<P><P>Hardware notes: In hardware this block costs nothing."  block_version=VER_STRING_GOES_HERE
  Up Sample{1..3,12,14,17..18} (7 alike) : xbsIndex_r4/Up Sample  sample_ratio=2  copy_samples=on  en=off  latency=0  dbl_ovrd=off  block_type=usamp
      settings: infoedit="Up samples input data.  Inserted values  can be zeros or copies of the most recent input sample.<P><P>Hardware notes: No hardware is needed if inserted values are copies of the input sample; otherwise, a mux and single bit flip-flop are used."  block_version=VER_STRING_GOES_HERE
  Up Sample{4..9,11,15..16,21} (10 alike) : xbsIndex_r4/Up Sample  sample_ratio=4  copy_samples=on  en=off  latency=0  dbl_ovrd=off  block_type=usamp
      settings: infoedit="Up samples input data.  Inserted values  can be zeros or copies of the most recent input sample.<P><P>Hardware notes: No hardware is needed if inserted values are copies of the input sample; otherwise, a mux and single bit flip-flop are used."  block_version=VER_STRING_GOES_HERE
  debug_chipscopeTrig1 : xbsIndex_r4/Gateway In  gui_display_data_type=Fixed-point  arith_type=Boolean  n_bits=14  bin_pt=13  preci_type=Single  exp_width=8  frac_width=24  quantization="Round  (unbiased: +/- Inf)"  overflow=Saturate  period=1  dbl_ovrd=off  timing_constraint=None  locs_specified=off  LOCs={}  inherit_from_input=off  UseAsADC=off  ADCChannel='1'  hdl_port=on  block_type=gatewayin
      settings: infoedit="Gateway in block.  Converts inputs of type Simulink integer, double and fixed point to  Xilinx fixed point type.<P><P>Hardware notes:  In hardware these blocks become top level input ports."  block_version=VER_STRING_GOES_HERE
  wires:
  [Payload] -> Up Sample11/1
  [AntA_ADC_I_Filt] -> Up Sample21/1
  [EQ_I] -> Up Sample17/1
  [EQ_Q] -> Up Sample18/1
  [Long_Corr] -> Slice1/1
  Constant1/1 -> debug_chipscopeTrig1/1
  Up Sample11/1 -> ChipScope1/1, ChipScope1/8  "Payload"
  Up Sample16/1 -> ChipScope1/15  "LongCorr"
  Up Sample17/1 -> ChipScope1/13  "EQ_I"
  Up Sample18/1 -> ChipScope1/14  "EQ_Q"
  Up Sample21/1 -> ChipScope1/11  "AntA_ADC_I"
  debug_chipscopeTrig1/1 -> ChipScope1/2  "ExtTrig"
  [intOut_GoodPkt] -> Up Sample12/1
  [intOut_BadPkt] -> Up Sample14/1
  Up Sample12/1 -> ChipScope1/3, ChipScope1/9  "Good Pkt Int"
  Up Sample14/1 -> ChipScope1/4, ChipScope1/10  "Bad Pkt Int"
  [AntA_ADC_Q_Filt] -> Up Sample15/1
  Up Sample15/1 -> ChipScope1/12  "AntA_ADC_Q"
  Counter1/1 -> Slice2/1
  Slice2/1 -> ChipScope1/5  "SampClk"
  [Chipscope_ChanEst] -> ChipScope1/17  "ChanEst"
  [PilotPhaseErr] -> ChipScope1/18  "PilotPhaseErr"
  Convert2/1 -> Up Sample16/1
  [intOut_GoodHeader] -> Up Sample2/1
  [intOut_BadHeader] -> Up Sample3/1
  [DebugOut_EVM] -> Convert1/1
  Up Sample1/1 -> ChipScope1/16  "EVM"
  Up Sample2/1 -> ChipScope1/19  "Good Header Int"
  Up Sample3/1 -> ChipScope1/20  "Bad Header Int"
  Up Sample4/1 -> ChipScope1/21  "PktDet"
  [pktDet] -> Up Sample4/1
  [AntA_AGC_Done] -> Up Sample5/1
  [AntA_GainBB] -> Up Sample6/1
  [AntA_GainRF] -> Up Sample7/1
  Up Sample5/1 -> ChipScope1/22  "AntA_AGC_Done"
  Up Sample6/1 -> ChipScope1/23  "AntA_GainBB"
  Up Sample7/1 -> ChipScope1/24  "AntA_GainRF"
  [FFT_Overflow] -> ChipScope1/25  "FFT_Overflow"
  Convert1/1 -> Up Sample1/1
  Slice1/1 -> Convert2/1
  [pktDet_rssi] -> Up Sample8/1
  Up Sample8/1 -> ChipScope1/26, ChipScope1/6  "pktDet_RSSI"
  [pktDet_corr] -> Up Sample9/1
  Up Sample9/1 -> ChipScope1/27, ChipScope1/7  "pktDet_Corr"
